simulink library "Renault_Library"  (Simulink 9.2)
settings: Solver=ode3  StartTime=0.0  StopTime=10.0  FixedStep=0.020  RelTol=1e-3  EnableMultiTasking=on
undefined symbols (not defined in the file or in its folder): ABC, AssystemProposal, b, chkval, CoeffA_C, CoeffB_C, CoeffMean_C, ConfTime_C, DiagDelay_C, DividePercent, FailureConfActive_C, FallingEdgeDuration_C, HighLevelThresholdVoltage_C, HighLevelVoltage_C, HighLevelVoltageTunable_C, InitValue_C, InputVoltRangeMax_C, InputVoltRangeMin_C, LowActiveContact_C, LowLevelThresholdVoltage_C, LowLevelVoltage_C, MaxOperatingVoltage_C, MinOperatingVoltage_C, mV2V_conversion, OUT1, OutOfRangeHigh_C, OutOfRangeLow_C, PinSupplySaturationMax, PinSupplySaturationMin, Res1Value_C, Res2Value_C, Res3Value_C, RisingEdgeDuration_C, RSA_CANSignalFailMonitoring, SampleTime_C, service14Table, service19Table, service22Table, service24Table, service28Table, service2ETable, service2FTable, service31Table, service87Table, SizeXStateDID2FTable, SizeXTable14, SizeXTable19, SizeXTable22, SizeXTable24, SizeXTable28, SizeXTable2E, SizeXTable2F, SizeXTable31, SizeXTable87, SizeYStateDID2FTable, SizeYTable14, SizeYTable19, SizeYTable22, SizeYTable24, SizeYTable28 ... (+22)
undefined symbols (not defined in the file): BatteryVoltage_ActivationThreshold_C, FrameStatus_AbsentValue_C, FrameStatus_ConfAbsentValue_C, MemThreshold_C, NAMEOFTHEMODEL_SAMPLE_TIME_CST, NbAbs1_C, NbConfAbs1_C, NbConfPres1_C, NbPres1_C, NOMDUSIGNAL_FaultConfTime_C, NOMDUSIGNAL_FaultRehabTime_C, NOMDUSIGNAL_switchvalue_C, NOMDUSIGNAL_unavailablevalue_C, PeriodFrame1_C, ResetTime_C, WakeUpTime_C
libraries: $bdroot x112, simulink x75
(part 1 of 32)
settings Model: Creator=a070814  LastModifiedBy=40005501

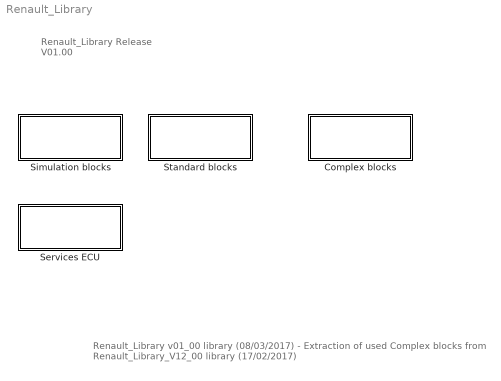
== system Renault_Library ==
  note: Renault_Library
      | Release V01.00
  note: Renault_Library v01_00 library (08/03/2017)
      | - Extraction of used Complex blocks from Renault_Library_V12_00 library (17/02/2017)
  Complex blocks : Subsystem  TreatAsAtomicUnit=on
  Services ECU : Subsystem  TreatAsAtomicUnit=on
  Simulation blocks : Subsystem  TreatAsAtomicUnit=on
  Standard blocks : Subsystem  TreatAsAtomicUnit=on

== system Renault_Library/Complex blocks ==
  note: Complex blocks
  Discontinuities : Subsystem  TreatAsAtomicUnit=on
  Discrete : Subsystem  TreatAsAtomicUnit=on
  Flip-Flop : Subsystem  TreatAsAtomicUnit=on
  Logic & Bit Operations : Subsystem  TreatAsAtomicUnit=on
  Lookup Tables : Subsystem  TreatAsAtomicUnit=on
  Math Operations : Subsystem  TreatAsAtomicUnit=on
  Signal Routing : Subsystem  TreatAsAtomicUnit=on
  Timers & Counters : Subsystem  TreatAsAtomicUnit=on

== system Renault_Library/Services ECU ==
  note: Services ECU
  Commonly_Used_Blocks : Subsystem
  Diag : Subsystem
  EEPROM : Subsystem
  HW Inputs : Subsystem
  HW Outputs : Subsystem
  Mux : Subsystem
  PowerSupply : Subsystem

== system Renault_Library/Simulation blocks ==
  note: Simulation
  DigitalClock : DigitalClock
  Display : Display
  FromFile : FromFile  SampleTime=0
  FromWorkspace : FromWorkspace  VariableName=simin  SampleTime=0
  ManualSwitch : simulink/Signal Routing/Manual Switch  SystemSampleTime=-1  sw=1  action=0  varsize=off
      settings: SourceProductName=Simulink  SourceProductBaseCode=SL
  PulseGenerator : DiscretePulseGenerator  PulseType=Time based  Period=10  PulseWidth=5
  Ramp : simulink/Sources/Ramp  SystemSampleTime=-1  slope=1  start=0  X0=0
  RateTransition : RateTransition
  RepeatingSequence : simulink/Sources/Repeating Sequence  SystemSampleTime=-1  rep_seq_t=[0 2]  rep_seq_y=[0 2]
  Scope : Scope
  SignalGenerator : SignalGenerator
  SineWave : Sin  SampleTime=0
  Step : Step  SampleTime=0
  Stop Simulation : Stop
  ToFile : ToFile  SaveFormat=Timeseries
  ToWorkspace : ToWorkspace  VariableName=simout  SaveFormat=Structure  SampleTime=-1

== system Renault_Library/Standard blocks ==
  note: Standard blocks
  Commonly Used Blocks : Subsystem  TreatAsAtomicUnit=on
  Discontinuities : Subsystem  TreatAsAtomicUnit=on
  Discrete : Subsystem  TreatAsAtomicUnit=on
  Logic & Bit Operations : Subsystem  TreatAsAtomicUnit=on
  Math Operations : Subsystem  TreatAsAtomicUnit=on
  Model Wide Utilities : Subsystem  TreatAsAtomicUnit=on
  Ports & Subsystems : Subsystem  TreatAsAtomicUnit=on
  Signal Attributes : Subsystem  TreatAsAtomicUnit=on
  Signal Routing : Subsystem  TreatAsAtomicUnit=on
  Sinks : Subsystem  TreatAsAtomicUnit=on
  Sources : Subsystem  TreatAsAtomicUnit=on

== system Renault_Library/Complex blocks/Discontinuities ==
  note: Discontinuities
  note: /!\         DO NOT USE THIS RED BLOCKS ANYMORE          /!\
      |
      |
      |
      |
      |
      |
      |
      |
      |
      |
      |
      |
      |
      |
      |
      |
      |
      |
      |
      |
      |
      |
      |
      |
      |
      |
      |
      |
      |
      |
      |
      |
      |
      |
      |
      |
      |
      |
      |
      |
      |
      |
      |
      |
      |
      |
      |
      |
      |
      |
      |
      |
      |
      |
      | .
  RSA_DetectionSaturation : Subsystem  Tag=[LIB]  mask(RSA_DetectionSaturation){}
      mask help:
      | Limit input signal to the upper and lower saturation values. Saturation of the [2](input) by entry [1] (maximum) and entry [3] (minimum). Output (sat) will be any of the following values
      |   0 : Input is within the threshold range
      |   1 : Input is less than minimum threshold
      |   2 : Input is greater than maximum threshold
      |   3 : The minimum threshold is equal to the maximum threshold.
      |
      | Version : 01.02
      mask init:
      | SATPAS = 0;
      | SATMIN = 1;
      | SATMAX = 2;
      settings: AttributesFormatString=%<MaskType> : Ver : 01.02
      mask Help: eval(['open([','''\\F-khepri\shared\DIM-DCMAP-CGMP\66161\Shared_MatLab\Tools_DCMAP\Common_Library_MBDR\Library\Common_Library\Help\RLV_RSA_DetectionSaturation_Spec_V1.02.pdf''','])'])
      mask Display: plot(0,0,100,100,[100,80,20,0],[80,80,20,20],[50,50],[0,100],[100,0],[50,50])
  RSA_Hysteresis : Subsystem  Tag=[LIB]  mask(RSA_Hysteresis){}
      mask help:
      | Aim of this function is to define two ranges for control relay. The Block switches the output from 0 to 1 when the input is greater than or equal to the High_Threshold, provided High_Threshold value is greater than the Low_Threshold.
      | The block switches the output from 1 to 0 when the input is less than or equal to Low_Threshold value, provided Low_Threshold value is less than the High_Threshold.
      |
      | Version : 01.02
      mask init:
      | HYSINI = 0;
      settings: AttributesFormatString=%<MaskType> : Ver : 01.02
      mask Help: eval(['open([','''\\F-khepri\shared\DIM-DCMAP-CGMP\66161\Shared_MatLab\Tools_DCMAP\Common_Library_MBDR\Library\Common_Library\Help\RLV_RSA_Hysteresis_Spec_V1.02.pdf''','])'])
      mask Display: disp(get_param(gcb,'name'))
  RSA_Hysteresis1 : Subsystem  Tag=[LIB]  mask(RSA_Hysteresis1){Th1_Strict=on, Th2_Strict=on}
      mask prompts: Th1_Strict "Output Value 1 when Inputfalls to Threshold 1"; Th2_Strict "Output Value 2 when Input increases to Threshold 2"
      mask help:
      | This block outputs 'Value 1' if 'Input' is lower than 'Threshold 1', and 'Value 2' if 'Input' is upper than 'Threshold 2'. Between these two values, there is an hysteresis.
      |
      | Version : 01.03
      mask init:
      | dot_x = [-0.02 -0.014 0 0.014 0.02 0.014 0 -0.014 -0.02];
      | dot_y = [0 0.014 0.02 0.014 0 -0.014 -0.02 -0.014 0];
      | Val1 = 0.2; 
      | Val2 = 0.82;
      | Open_T1 = dot_y + Val2;
      | Closed_T1 = dot_y + Val1;
      | Open_T2 = dot_y + Val1;
      | Closed_T2 = dot_y + Val2-0.01;
      | Path_y = [Val1 Val1 Val2 Val2];
      | 
      | if(Th1_Strict)
      |     Th1_falling='V1';
      |     Th1_V1 = 1;
      |     Th1_V2 = 0;
      |     Open_T1 = dot_y + Val2;
      |     Closed_T1 = dot_y + Val1;
      |     Fall_path_x = [0.2 0.4 0.43 0.8];
      |     Fall_arrow_x = [0.39 0.41 0.43];
      | else
      |     Th1_falling='V2';
      |     Th1_V1 = 0;
      |     Th1_V2 = 1;
      |     Open_T1 = dot_y + Val1;
      |     Closed_T1 = dot_y + Val2-0.01;
      |     Fall_path_x = [0.2 0.37 0.4 0.8];
      |     Fall_arrow_x = [0.36 0.38 0.40];
      | end
      | if(Th2_Strict)
      |     Th2_rising='V2';
      |     Th2_V1 = 0;
      |     Th2_V2 = 1;
      |     Open_T2 = dot_y + Val1;
      |     Closed_T2 = dot_y + Val2-0.01;
      |     Rise_path_x = [0.2 0.57 0.6 0.8];
      |     Rise_arrow_x = [0.57 0.59 0.61];
      | else
      |     Th2_rising='V1';
      |     Th2_V1 = 1;
      |     Th2_V2 = 0;
      |     Open_T2 = dot_y + Val2;
      |     Closed_T2 = dot_y + Val1;
      |     Rise_path_x = [0.23 0.6 0.63 0.8];
      |     Rise_arrow_x = [0.6 0.62 0.64];
      | end
      settings: AttributesFormatString=%<MaskType> : Ver : 01.03\nOutput Value 1 when Input falls to Threshold 1: %<Th1_Strict>\nOutput Value 2 when Input increases to Threshold 2: %<Th2_Strict>
      mask Help: eval(['open([','''\\F-khepri\shared\DIM-DCMAP-CGMP\66161\Shared_MatLab\Tools_DCMAP\Common_Library_MBDR\Library\Common_Library\Help\RLV_RSA_Hysteresis1_Spec_V1.03.pdf''','])'])
      mask Display:
      | disp(['RSA_hysteresis' char(10) char(10) char(10) char(10) char(10) char(10) char(10) char(10) char(10)])
      | 
      | plot(Fall_path_x,Path_y,Rise_path_x,Path_y)
      | 
      | plot(Fall_arrow_x,[0.45 0.4 0.45])
      | plot(Rise_arrow_x,[0.55 0.6 0.55])
      | 
      | plot(dot_x+0.4,Open_T1)
      | patch(dot_x+0.4,Closed_T1,[0 0 0])
      | 
      | plot(dot_x+0.6,Open_T2)
      | patch(dot_x+0.6,Closed_T2,[0 0 0])
      | 
      | disp([char(10) char(10)   '         T1      T2' char(10) char(10)])
      | disp([ char(10) char(10) char(10) char(10) char(10) ' v1                           '])
      | disp(['                           v2'  char(10) char(10) char(10) char(10) char(10) char(10) char(10)])
  RSA_Hysteresis1_v01_04 : Subsystem  Tag=[LIB]  mask(RSA_Hysteresis1){Th1_Strict=on, Th2_Strict=on}
      mask prompts: Th1_Strict "Output Value 1 when Inputfalls to Threshold 1"; Th2_Strict "Output Value 2 when Input increases to Threshold 2"
      mask help:
      | This block outputs 'Value 1' if 'Input' is lower than 'Threshold 1', and 'Value 2' if 'Input' is upper than 'Threshold 2'. Between these two values, there is an hysteresis. When both the parameters are cleared, if the input is upper than 'Threshold 2' then the output will be 'Value 2' otherwise it maintains the previous value.
      |
      | Version : 01.04
      mask init: same as Renault_Library/Complex blocks/Discontinuities/RSA_Hysteresis1
      settings: AttributesFormatString=%<MaskType> : Ver : 01.04\nOutput Value 1 when Input falls to Threshold 1: %<Th1_Strict>\nOutput Value 2 when Input increases to Threshold 2: %<Th2_Strict>
      mask Help: eval(['open([','''\\F-khepri\shared\DIM-DCMAP-CGMP\66161\Shared_MatLab\Tools_DCMAP\Common_Library_MBDR\Library\Common_Library\Help\RLV_RSA_Hysteresis1_Spec_V1.04.pdf''','])'])
      mask Display:
      | disp(['RSA_hysteresis' char(10) char(10) char(10) char(10) char(10) char(10) char(10) char(10) char(10)])
      | 
      | plot(Fall_path_x,Path_y,Rise_path_x,Path_y)
      | 
      | plot(Fall_arrow_x,[0.45 0.4 0.45])
      | plot(Rise_arrow_x,[0.55 0.6 0.55])
      | 
      | plot(dot_x+0.4,Open_T1)
      | patch(dot_x+0.4,Closed_T1,[0 0 0])
      | 
      | plot(dot_x+0.6,Open_T2)
      | patch(dot_x+0.6,Closed_T2,[0 0 0])
      | 
      | disp([char(10) char(10)   '         T1      T2' char(10) char(10)])
      | disp([ char(10) char(10) char(10) char(10) char(10) ' v1                           '])
      | disp(['                           v2'  char(10) char(10) char(10) char(10) char(10) char(10) char(10)])
  RSA_Hysteresis1_v01_05 : Subsystem  Tag=[LIB]  mask(RSA_Hysteresis1){Th1_Strict=on, Th2_Strict=off}
      mask prompts: Th1_Strict "Output Value 1 when Inputfalls to Threshold 1"; Th2_Strict "Output Value 2 when Input increases to Threshold 2"
      mask help:
      | This block outputs 'Value 1' if 'Input' is lower than 'Threshold 1', and 'Value 2' if 'Input' is upper than 'Threshold 2'. Between these two values, there is an hysteresis. When both the parameters are cleared, if the input is upper than 'Threshold 2' then the output will be 'Value 2' otherwise it maintains the previous value.
      |
      | Version : 01.05
      mask init:
      | dot_x = [-0.02 -0.014 0 0.014 0.02 0.014 0 -0.014 -0.02];
      | dot_y = [0 0.014 0.02 0.014 0 -0.014 -0.02 -0.014 0];
      | Val1 = 0.2; 
      | Val2 = 0.82;
      | Open_T1 = dot_y + Val2;
      | Closed_T1 = dot_y + Val1;
      | Open_T2 = dot_y + Val1;
      | Closed_T2 = dot_y + Val2-0.01;
      | Path_y = [Val1 Val1 Val2 Val2];
      | 
      | if(Th1_Strict)
      |     Th1_falling='V1';
      |     Th1_V1 = 1;
      |     Th1_V2 = 0;
      |     set_param([gcb '/RO_Th1Strict'],'Operator','>=');
      |     Open_T1 = dot_y + Val2;
      |     Closed_T1 = dot_y + Val1;
      |     Fall_path_x = [0.2 0.4 0.43 0.8];
      |     Fall_arrow_x = [0.39 0.41 0.43];
      | else
      |     Th1_falling='V2';
      |     Th1_V1 = 0;
      |     Th1_V2 = 1;
      |     set_param([gcb '/RO_Th1Strict'],'Operator','>');
      |     Open_T1 = dot_y + Val1;
      |     Closed_T1 = dot_y + Val2-0.01;
      |     Fall_path_x = [0.2 0.37 0.4 0.8];
      |     Fall_arrow_x = [0.36 0.38 0.40];
      | end
      | if(Th2_Strict)
      |     Th2_rising='V2';
      |     Th2_V1 = 0;
      |     Th2_V2 = 1;
      |      set_param([gcb '/RO_Th2Strict'],'Operator','>=');
      |     Open_T2 = dot_y + Val1;
      |     Closed_T2 = dot_y + Val2-0.01;
      |     Rise_path_x = [0.2 0.57 0.6 0.8];
      |     Rise_arrow_x = [0.57 0.59 0.61];
      | else
      |     Th2_rising='V1';
      |     Th2_V1 = 1;
      |     Th2_V2 = 0;
      |     set_param([gcb '/RO_Th2Strict'],'Operator','>');
      |     Open_T2 = dot_y + Val2;
      |     Closed_T2 = dot_y + Val1;
      |     Rise_path_x = [0.23 0.6 0.63 0.8];
      |     Rise_arrow_x = [0.6 0.62 0.64];
      | end
      settings: AttributesFormatString=%<MaskType> : Ver : 01.05\nOutput Value 1 when Input falls to Threshold 1: %<Th1_Strict>\nOutput Value 2 when Input increases to Threshold 2: %<Th2_Strict>
      mask Help: eval(['open([','''\\F-khepri\shared\DIM-DCMAP-CGMP\66161\Shared_MatLab\Tools_DCMAP\Common_Library_MBDR\Library\Common_Library\Help\RLV_RSA_Hysteresis1_Spec_V1.05.pdf''','])'])
      mask Display:
      | disp(['RSA_hysteresis' char(10) char(10) char(10) char(10) char(10) char(10) char(10) char(10) char(10)])
      | 
      | plot(Fall_path_x,Path_y,Rise_path_x,Path_y)
      | 
      | plot(Fall_arrow_x,[0.45 0.4 0.45])
      | plot(Rise_arrow_x,[0.55 0.6 0.55])
      | 
      | plot(dot_x+0.4,Open_T1)
      | patch(dot_x+0.4,Closed_T1,[0 0 0])
      | 
      | plot(dot_x+0.6,Open_T2)
      | patch(dot_x+0.6,Closed_T2,[0 0 0])
      | 
      | disp([char(10) char(10)   '         T1      T2' char(10) char(10)])
      | disp([ char(10) char(10) char(10) char(10) char(10) ' v1                           '])
      | disp(['                           v2'  char(10) char(10) char(10) char(10) char(10) char(10) char(10)])
  RSA_Hysteresis_V01_03 : Subsystem  Tag=[LIB]  mask(RSA_Hysteresis){}
      mask help:
      | Aim of this function is to define two ranges for control relay. The Block switches the output from 0 to 1 when the input is greater than or equal to the High_Threshold, provided High_Threshold value is greater than the Low_Threshold.
      | The block switches the output from 1 to 0 when the input is less than or equal to Low_Threshold value, provided Low_Threshold value is less than the High_Threshold.
      |
      | Version : 01.03
      mask init:
      | HYSINI = 0;
      settings: AttributesFormatString=%<MaskType> : Ver : 01.03
      mask Help: eval(['open([','''\\F-khepri\shared\DIM-DCMAP-CGMP\66161\Shared_MatLab\Tools_DCMAP\Common_Library_MBDR\Library\Common_Library\Help\RLV_RSA_Hysteresis_Spec_V1.03.pdf''','])'])
      mask Display: disp(get_param(gcb,'name'))
  RSA_Hysteresis_WithConf_V01_00 : Subsystem  Tag=[LIB]  mask(RSA_Hysteresis_WithConfirmation){Th1_Strict=on, Th2_Strict=off, int_dt=on}
      mask prompts: Th1_Strict "Output Value 1 when Inputfalls to Threshold 1"; Th2_Strict "Output Value 2 when Input increases to Threshold 2"; int_dt "Tick if datatype of Confirmation time is integer with resolution 1"
      mask help:
      | This block outputs 'Value 1' if 'Input' is lower than 'Threshold 1' during the 'ConfirmationTime', and 'Value 2' if 'Input' is upper than 'Threshold 2' during the 'ConfirmationTime'. Between these two values, there is an hysteresis. When both the parameters are cleared, if the input is upper than 'Threshold 2' then the output will be 'Value 2' otherwise it maintains the previous value.
      |
      | Version : 01.00
      mask init:
      | dot_x = [-0.02 -0.014 0 0.014 0.02 0.014 0 -0.014 -0.02];
      | dot_y = [0 0.014 0.02 0.014 0 -0.014 -0.02 -0.014 0];
      | Val1 = 0.2; 
      | Val2 = 0.82;
      | Open_T1 = dot_y + Val2;
      | Closed_T1 = dot_y + Val1;
      | Open_T2 = dot_y + Val1;
      | Closed_T2 = dot_y + Val2-0.01;
      | Path_y = [Val1 Val1 Val2 Val2];
      | 
      | TIM1INI = 0;
      | CONS_ONE =1;
      | CONS_ZERO=0;
      | BOOSTING_FLP=1000;
      | BOOSTING_FXP=1;
      | timeoutinit=0;
      | initinit=0;
      | 
      | 
      | if strcmp(get_param(gcb,'int_dt'),'on')
      |     set_param([gcb '/TurnOnDelay1/From11'],'GotoTag','CONS_ONE');
      |     set_param([gcb '/TurnOnDelay1/From13'],'GotoTag','CONS_ONE');
      |     set_param([gcb '/TurnOnDelay2/From11'],'GotoTag','CONS_ONE');
      |     set_param([gcb '/TurnOnDelay2/From13'],'GotoTag','CONS_ONE');
      | else
      |     set_param([gcb '/TurnOnDelay1/From11'],'GotoTag','tolcons');
      |     set_param([gcb '/TurnOnDelay1/From13'],'GotoTag','tolcons');
      |     set_param([gcb '/TurnOnDelay2/From11'],'GotoTag','tolcons');
      |     set_param([gcb '/TurnOnDelay2/From13'],'GotoTag','tolcons');
      | end
      | 
      | if(Th1_Strict)
      |     Th1_falling='V1';
      |     Th1_V1 = 1;
      |     Th1_V2 = 0;
      |     set_param([gcb '/RO_Th1Strict'],'Operator','>=');
      |     Open_T1 = dot_y + Val2;
      |     Closed_T1 = dot_y + Val1;
      |     Fall_path_x = [0.2 0.4 0.43 0.8];
      |     Fall_arrow_x = [0.39 0.41 0.43];
      | else
      |     Th1_falling='V2';
      |     Th1_V1 = 0;
      |     Th1_V2 = 1;
      |     set_param([gcb '/RO_Th1Strict'],'Operator','>');
      |     Open_T1 = dot_y + Val1;
      |     Closed_T1 = dot_y + Val2-0.01;
      |     Fall_path_x = [0.2 0.37 0.4 0.8];
      |     Fall_arrow_x = [0.36 0.38 0.40];
      | end
      | if(Th2_Strict)
      |     Th2_rising='V2';
      |     Th2_V1 = 0;
      |     Th2_V2 = 1;
      |      set_param([gcb '/RO_Th2Strict'],'Operator','>=');
      |     Open_T2 = dot_y + Val1;
      |     Closed_T2 = dot_y + Val2-0.01;
      |     Rise_path_x = [0.2 0.57 0.6 0.8];
      |     Rise_arrow_x = [0.57 0.59 0.61];
      | else
      |     Th2_rising='V1';
      |     Th2_V1 = 1;
      |     Th2_V2 = 0;
      |     set_param([gcb '/RO_Th2Strict'],'Operator','>');
      |     Open_T2 = dot_y + Val2;
      |     Closed_T2 = dot_y + Val1;
      |     Rise_path_x = [0.23 0.6 0.63 0.8];
      |     Rise_arrow_x = [0.6 0.62 0.64];
      | end
      settings: AttributesFormatString=|
      | %<MaskType> : Ver : 01.00\nOutput Value 1 when Input falls to Threshold 1: %<Th1_Strict>\nOutput Value 2 when Input increases to Threshold 2: %<Th2_Strict>
      | Confirmation Time of integer datatype with resolution 1 : "%<int_dt>"
      mask Help: eval(['open([','''\\F-khepri\shared\DIM-DCMAP-CGMP\66161\Shared_MatLab\Tools_DCMAP\Common_Library_MBDR\Library\Common_Library\Help\RLV_RSA_Hysteresis_WithConf_Spec_V1.00.pdf''','])'])
      mask Display:
      | disp(['RSA_hysteresis' char(10) char(10) char(10) char(10) char(10) char(10) char(10) char(10) char(10)])
      | 
      | plot(Fall_path_x,Path_y,Rise_path_x,Path_y)
      | 
      | plot(Fall_arrow_x,[0.45 0.4 0.45])
      | plot(Rise_arrow_x,[0.55 0.6 0.55])
      | 
      | plot(dot_x+0.4,Open_T1)
      | patch(dot_x+0.4,Closed_T1,[0 0 0])
      | plot(dot_x+0.35,Open_T1-0.68)
      | plot([dot_x(3),dot_x(5)]+0.35,[Open_T1(3)-0.02,Open_T1(5)]-0.68)
      | plot([dot_x(3),dot_x(3)+(dot_x(4)-dot_x(3))/2]+0.35,[Open_T1(3)-0.02,(Open_T1(3)-0.02)+(Open_T1(4)-Open_T1(3)+0.02)/2]-0.68)
      | 
      | plot(dot_x+0.6,Open_T2)
      | plot(dot_x+0.65,Open_T2+0.05)
      | plot([dot_x(3),dot_x(5)]+0.65,[Open_T1(3)-0.02,Open_T1(5)]+0.05)
      | plot([dot_x(3),dot_x(3)+(dot_x(4)-dot_x(3))/2]+0.65,[Open_T1(3)-0.02,(Open_T1(3)-0.02)+(Open_T1(4)-Open_T1(3)+0.02)/2]+0.05)
      | patch(dot_x+0.6,Closed_T2,[0 0 0])
      | 
      | disp([char(10) char(10)   '         T1      T2' char(10) char(10)])
      | disp([ char(10) char(10) char(10) char(10) char(10) ' v1                           '])
      | disp(['                           v2'  char(10) char(10) char(10) char(10) char(10) char(10) char(10)])
  RSA_MaxSaturation : Subsystem  Tag=[LIB]  mask(RSA_MaxSaturation){Max=2.1}
      mask prompts: Max "Upper limit"
      mask help:
      | Limits the signal to the upper value specified.
      |
      | Version : 01.02
      settings: AttributesFormatString=%<MaskType> : Ver : 01.02\nUpper limit %<Max>
      mask Help: eval(['open([','''\\F-khepri\shared\DIM-DCMAP-CGMP\66161\Shared_MatLab\Tools_DCMAP\Common_Library_MBDR\Library\Common_Library\Help\RLV_RSA_MaxSaturation_Spec_V1.02.pdf''','])'])
      mask Display: % disp('MaxSat')
  RSA_MinSaturation : Subsystem  Tag=[LIB]  mask(RSA_MinSaturation){Min=2.1}
      mask prompts: Min "Lower limit"
      mask help:
      | Limits signal to the lower value specified.
      |
      | Version : 01.02
      settings: AttributesFormatString=%<MaskType> : Ver : 01.02\nLower limit %<Min>
      mask Help: eval(['open([','''\\F-khepri\shared\DIM-DCMAP-CGMP\66161\Shared_MatLab\Tools_DCMAP\Common_Library_MBDR\Library\Common_Library\Help\RLV_RSA_MinSaturation_Spec_V1.02.pdf''','])'])
      mask Display: % disp('MinSat')

== system Renault_Library/Complex blocks/Discrete ==
  note: Discrete
  note: /!\         DO NOT USE THIS RED BLOCKS ANYMORE          /!\
      |
      |
      |
      |
      |
      |
      |
      |
      |
      |
      |
      |
      |
      |
      |
      |
      |
      |
      |
      |
      |
      |
      |
      |
      |
      |
      |
      |
      |
      |
      |
      |
      |
      |
      |
      |
      |
      |
      | .
  RSA_TappedDelay : Subsystem  Tag=[LIB]  mask(RSA_TappedDelay){NoOfDelayS=2, OrderValue=Oldest, SampleTime=-1, InitialValue=0.9, AppendValue=off}
      mask prompts: NoOfDelayS "No Of Delay"; OrderValue "Order output vector starting with"; SampleTime "Sample Time"; InitialValue "Initial condition"; AppendValue "Include current input in output vector"
      mask help:
      | Delay a signal by 'N' sample periods and output all the delayed versions of the signal.
      |
      | Version : 01.02
      mask init:
      | INIADD = 1;
      | TAPDINI = 0;
      | INIVECSEL = 2;
      | 
      | if NoOfDelayS==-1
      |     NoOfDelay=1;
      | else
      |     NoOfDelay=NoOfDelayS;
      | end
      | A_NoOfDelay=1:NoOfDelay;
      | if NoOfDelay==1
      | Selector=1:NoOfDelay;    
      | 
      | else
      | Selector=1:NoOfDelay-1;    
      | end
      | Append = (2-AppendValue):NoOfDelay+1;
      | Order=OrderValue-1;
      | 
      | if AppendValue
      | Order_Selec_Req=1:(NoOfDelay+1);
      | Order_Selec_Inv = (NoOfDelay+1):-1:1;
      | 
      | else 
      | 
      | Order_Selec_Req=1:NoOfDelay;
      | Order_Selec_Inv = NoOfDelay:-1:1;
      | 
      | end
      settings: AttributesFormatString=%<MaskType> : Ver : 01.02\nNo Of Delay %<NoOfDelayS>\nOrder output vector starting with %<OrderValue>\nInitial condition %<InitialValue>\nInclude current input in output vector %<AppendValue>
      mask Help: eval(['open([','''\\F-khepri\shared\DIM-DCMAP-CGMP\66161\Shared_MatLab\Tools_DCMAP\Common_Library_MBDR\Library\Common_Library\Help\RLV_RSA_TappedDelay_Spec_V1.02.pdf''','])'])
      mask Callback NoOfDelayS:
      | A = str2num(get_param(gcb,'NoOfDelayS'));
      | if (A<=0&&A~=-1)
      |     errorString= ['At: ' gcb '. The number of delays must be positive or -1 for inherted.']
      |     errordlg(errorString)
      | end
      mask TypeOptions OrderValue: Newest | Oldest
      mask Display: fprintf('%d\nDelays',NoOfDelayS);
  RSA_TappedDelay_V01_03 : Subsystem  Tag=[LIB]  mask(RSA_TappedDelay){NoOfDelayS=4, OrderValue=Newest, SampleTime=-1, InitialValue=5, AppendValue=off}
      mask prompts: NoOfDelayS "No Of Delay"; OrderValue "Order output vector starting with"; SampleTime "Sample Time"; InitialValue "Initial condition"; AppendValue "Include current input in output vector"
      mask help:
      | Delay a signal by 'N' sample periods and output all the delayed versions of the signal.
      |
      | Version : 01.03
      mask init:
      | INIADD = 1;
      | 
      | TAPDINI = 0;
      | % INIVECSEL = 2;
      | 
      | if NoOfDelayS==-1
      |     NoOfDelay=1;
      | else
      |     NoOfDelay=NoOfDelayS;
      | end
      | A_NoOfDelay=1:NoOfDelay;
      | if NoOfDelay==1
      | Selector=1:NoOfDelay;    
      | 
      | else
      | Selector=1:NoOfDelay-1;    
      | end
      | Append = (2-AppendValue):NoOfDelay+1;
      | if OrderValue==1
      |    set_param([gcb '/varOrder'],'GotoTag','newest_order'); 
      | else
      |    set_param([gcb '/varOrder'],'GotoTag','oldest_order'); 
      | end
      | % Order=OrderValue-1;
      | if AppendValue
      | Order_Selec_Req=1:(NoOfDelay+1);
      | Order_Selec_Inv = (NoOfDelay+1):-1:1;
      | else 
      | Order_Selec_Req=1:NoOfDelay;
      | Order_Selec_Inv = NoOfDelay:-1:1;
      | end
      settings: AttributesFormatString=|
      | %<MaskType> : Ver : 01.03
      | No Of Delay :%<NoOfDelayS>
      | Order output vector starting with :%<OrderValue>
      | SampleTime :%<SampleTime>
      | Initial condition :%<InitialValue>
      | Include current input in output vector :%<AppendValue>
      mask Help: eval(['open([','''\\F-khepri\shared\DIM-DCMAP-CGMP\66161\Shared_MatLab\Tools_DCMAP\Common_Library_MBDR\Library\Common_Library\Help\RLV_RSA_TappedDelay_Spec_V1.03.pdf''','])'])
      mask Callback NoOfDelayS:
      | A = str2num(get_param(gcb,'NoOfDelayS'));
      | if (A<=0&&A~=-1)
      |     errorString= ['At: ' gcb '. The number of delays must be positive or -1 for inherited.']
      |     errordlg(errorString)
      | end
      mask TypeOptions OrderValue: Newest | Oldest
      mask Display: fprintf('%d\nDelays',NoOfDelayS);
  RSA_UnitDelayExternalIC : Subsystem  Tag=[LIB]  mask(RSA_UnitDelayExternalIC){}
      mask help:
      | Normally, the output is the signal u delayed by one sample period.
      | The initial condition is given by the signal IC.
      |
      | Version : 01.02
      mask init:
      | UDEIC_zero = 0;
      settings: AttributesFormatString=%<MaskType> : Ver : 01.02
      mask Help: eval(['open([','''\\F-khepri\shared\DIM-DCMAP-CGMP\66161\Shared_MatLab\Tools_DCMAP\Common_Library_MBDR\Library\Common_Library\Help\RLV_RSA_UnitDelayExternalIC_Spec_V1.02.pdf''','])'])
      mask Display: dpoly(1,[1 0],'z')
  RSA_UnitDelayExternalIC_v01_03 : Subsystem  Tag=[LIB]  mask(RSA_UnitDelayExternalIC){}
      mask help:
      | Normally, the output is the signal u delayed by one sample period.
      | The initial condition is given by the signal IC.
      |
      | Version : 01.03
      mask init:
      | UDEIC_zero = 0;
      settings: AttributesFormatString=%<MaskType> : Ver : 01.03
      mask Help: eval(['open([','''\\F-khepri\shared\DIM-DCMAP-CGMP\66161\Shared_MatLab\Tools_DCMAP\Common_Library_MBDR\Library\Common_Library\Help\RLV_RSA_UnitDelayExternalIC_Spec_V1.03.pdf''','])'])
      mask Display: dpoly(1,[1 0],'z')

== system Renault_Library/Complex blocks/Flip-Flop ==
  note: Flip-Flop
  note: /!\         DO NOT USE THIS RED BLOCKS ANYMORE          /!\
      |
      |
      |
      |
      |
      |
      |
      |
      |
      |
      |
      |
      |
      |
      |
      | .
  RSA_SRFlipFlopSetPrioritary : Subsystem  Tag=[LIB]  mask(RSA_SRFlipFlopSetPrioritary){init=true}
      mask prompts: init "Initial condition (state of Q):"
      mask help:
      | This block performs the normal SR flip flop operation. But the input 'S'(Set) is having the high priority when both inputs (S,R) are TRUE. Following is the truth table for this block.
      |
      |  S  R  Q
      |  --------
      |  0  0   Q
      |  0  1   0
      |  1  0   1
      |  1  1   1
      |
      | Version : 01.02
      settings: AttributesFormatString=%<MaskType> : Ver : 01.02\n<init=%<init>>
      mask Help: eval(['open([','''\\F-khepri\shared\DIM-DCMAP-CGMP\66161\Shared_MatLab\Tools_DCMAP\Common_Library_MBDR\Library\Common_Library\Help\RLV_RSA_SRFlipFlopSetPrioritary_Spec_V1.02.pdf''','])'])
  RSA_SRFlipFlopSetPrioritary_v01_03 : Subsystem  Tag=[LIB]  mask(RSA_SRFlipFlopSetPrioritary){init=false}
      mask prompts: init "Initial condition (state of Q):"
      mask help:
      | This block performs the normal SR flip flop operation. But the input 'S'(Set) is having the high priority when both inputs (S,R) are TRUE. Following is the truth table for this block.
      |
      |  S  R  Q
      |  --------
      |  0  0   Q
      |  0  1   0
      |  1  0   1
      |  1  1   1
      |
      | WARNING: THIS BLOCK HAS BECOME OBSOLETE. PLEASE USE RSA_SRFlipFlop_V01_03 WITH "Output if Indeterminate Condition(When S = TRUE and R =TRUE)" PARAMETER AS "True"!!!
      |
      |
      | Version : 01.03
      settings: AttributesFormatString=%<MaskType> : Ver : 01.03\n<init=%<init>>
      mask Help: eval(['open([','''\\F-khepri\shared\DIM-DCMAP-CGMP\66161\Shared_MatLab\Tools_DCMAP\Common_Library_MBDR\Library\Common_Library\Help\RLV_RSA_SRFlipFlopSetPrioritary_Spec_V1.03.pdf''','])'])
  RSA_SRFlipFlop_v01_02 : Subsystem  Tag=[LIB]  mask(RSA_SRFlipFlop){IC=false}
      mask prompts: IC "Initial Condition"
      mask help:
      | The S-R flip-flop also known as a set-reset flip-flop. This implementation
      | is modelled after an S-R flip-flop constructed using NOR gates. The
      | truth table for this block is:
      |
      |  Set  ReSet    Out
      |  --------------------
      |  0      0         Out
      |  0      1         0
      |  1      0         1
      |  1      1         0
      |
      | In an S-R flip-flop that is implemented with NOR gates, when value 1 is
      | applied to both the set and reset inputs, output goes to 0. Technically,
      | this state is undefined and is usually avoided.
      | The initial value for the output is given by the mask parameter 'Initial Condition'.
      |
      | Version : 01.02
      settings: AttributesFormatString=%<MaskType> : Ver : 01.02\nInitial Condition = %<IC>
      mask Help: eval(['open([','''\\F-khepri\shared\DIM-DCMAP-CGMP\66161\Shared_MatLab\Tools_DCMAP\Common_Library_MBDR\Library\Common_Library\Help\RLV_RSA_SRFlipFlop_Spec_V1.02.pdf''','])'])
      mask Display: % disp(get_param(gcb,'name'))
  RSA_SRFlipFlop_v01_03 : Subsystem  Tag=[LIB]  mask(RSA_SRFlipFlop){Init=false, Indeterminate_Condition_out=false}
      mask prompts: Init "Initial Condition"; Indeterminate_Condition_out "Output if Indeterminate Condition (When S=TRUE and R=TRUE)"
      mask help:
      | The S-R flip-flop also known as a set-reset flip-flop. This implementation
      | is modelled after a S-R flip-flop constructed using Logic gates. The
      | truth table for this block is:
      |
      |  Set  ReSet    Out
      |  --------------------
      |  0      0    INIT VALUE OR PREV OUTPUT
      |  0      1        0
      |  1      0        1
      |  1      1    Indeterminate Value
      |
      | When value true is applied to both the SET(S) and RESET(R) inputs, output is set to "Output if Indeterminate Condition (When S=TRUE and R=TRUE)".
      | Ideally, this state is undefined and is usually avoided.
      | The initial value for the output is given by the mask parameter 'Initial Condition'.
      |
      | Version : 01.03
      mask init:
      | switch Init
      |     case 1
      |         IC = true;
      |     case 2
      |         IC = false;
      | end
      |     
      | switch Indeterminate_Condition_out
      |     case 1
      |         Indeterminate_Condition = true;
      |     case 2
      |         Indeterminate_Condition = false;
      | end
      settings: AttributesFormatString=|
      | %<MaskType> : Ver : 01.03
      | Initial Condition : %<Init>
      | Output when S=1 & R=1 : %<Indeterminate_Condition_out>
      mask Help: eval(['open([','''\\F-khepri\shared\DIM-DCMAP-CGMP\66161\Shared_MatLab\Tools_DCMAP\Common_Library_MBDR\Library\Common_Library\Help\RLV_RSA_SRFlipFlop_Spec_V1.03.pdf''','])'])
      mask TypeOptions Init: true | false
      mask TypeOptions Indeterminate_Condition_out: true | false
      mask Display: % disp(get_param(gcb,'name'))
